simulink model "BaseEngineController_A02_bak"  (Simulink 7.7)
settings: Solver=FixedStepDiscrete  StartTime=0.0  StopTime=10.0  RelTol=1e-3
PreLoadFcn:
  | try, eval([bdroot '_setup']); catch, eval('model_setup', ''); end
InitFcn:
  | try, eval([bdroot '_setup']); catch, eval('model_setup', ''); end
undefined symbols (not defined in the file or in its folder): ECU555, High, motohawk_build_version, motohawk_get_faults, motohawk_trunc_name, motohawk_vardec_config_group, motohawk_vardec_path, True
libraries: MotoHawk_lib x1145, AutoDoc_lib x786, motohawk_control_primitives_lib x114, motohawk_engine_lib x19, motohawk_annotations_lib x12, simulink x11, simulink_extras x1
(part 4 of 23)

== system BaseEngineController_A02_bak/Foreground/Control/ActuatorCharacterization/Injector Characterization ==
  FPC [mg] : Inport
  FuelMult : Inport  Port=2
  BaroPress [kPa] : Inport  Port=3
  MAP [kPa] : Inport  Port=4
  SysVolt09Idx : Inport  Port=5
  RPM : Inport  Port=6
  Constant : Constant  Value=60*1000*2
  DeltaPressureIndex : Subsystem
  Divide : Product  Inputs=*/
  Documentation_Calibrations_Probes_Block : AutoDoc_lib/Documentation_Calibrations_Probes_Block
  Documentation_Left_Border : AutoDoc_lib/Documentation_Left_Border  layout=Portrait
  Documentation_Right_Border : AutoDoc_lib/Documentation_Right_Border  layout=Portrait
  Documentation_Title_Block : AutoDoc_lib/Documentation_Title_Block
  Fuel Inj Offsets : Subsystem
  Injector Gain [ms/mg] : MotoHawk_lib/Calibration & Probing Blocks/motohawk_calibration  nam='InjGain'  val=0.351  storage=Calibration  show_more=on  name_source=Use Parameter  data_type=Inherit from 'Default Value'  read_level=1  write_level=1  ovr_cals=on  view_as=Number  enum_struct={'Disabled', 'Enabled'}  vector_style=Wide Row  use_help_units=on  help='Gain value used in the linear transformation of FPC to mechanical pulsewidth.'  units='ms/mg'  use_header_enum=off  row_header_enum={}  col_header_enum={}  use_min_max=off  min_val=0  max_val=inf  use_precision=off  precision='2.4'  gain=1  offset=0  exp=1  use_mototune_group=on  mototune_group='Actuators | Injectors | Injector Config'
  Injector Offset [ms] : MotoHawk_lib/Calibration & Probing Blocks/motohawk_calibration  nam='InjOffset'  val=-0.05  storage=Calibration  show_more=on  name_source=Use Parameter  data_type=Inherit from 'Default Value'  read_level=1  write_level=1  ovr_cals=on  view_as=Number  enum_struct={'Disabled', 'Enabled'}  vector_style=Wide Row  use_help_units=on  help='Offset value used in the linear transformation of FPC to mechanical pulsewidth.'  units='ms'  use_header_enum=off  row_header_enum={}  col_header_enum={}  use_min_max=off  min_val=-inf  max_val=inf  use_precision=off  precision='3.4'  gain=1  offset=0  exp=1  use_mototune_group=on  mototune_group='Actuators | Injectors | Injector Config'
  MinMax : MinMax  Inputs=2
  Mux1 : Mux  Inputs=3
  Product2 : Product  SaturateOnIntegerOverflow=on  InputSameDT=on  OutDataTypeStr=Inherit: Same as first input
  Product3 : Product  Inputs=3  SaturateOnIntegerOverflow=on  InputSameDT=on  OutDataTypeStr=Inherit: Same as first input
  Regulated Gauge Fuel Pressure [kPa] : MotoHawk_lib/Calibration & Probing Blocks/motohawk_calibration  nam='GaugeFuelPress'  val=343  storage=Calibration  show_more=on  name_source=Use Parameter  data_type=Inherit from 'Default Value'  read_level=1  write_level=1  ovr_cals=on  view_as=Number  enum_struct={'Disabled', 'Enabled'}  vector_style=Wide Row  use_help_units=on  help='Fuel pressure of fuel rail.'  units='kPa'  use_header_enum=off  row_header_enum={}  col_header_enum={}  use_min_max=on  min_val=0  max_val=inf  use_precision=off  precision=''  gain=1  offset=0  exp=1  use_mototune_group=on  mototune_group='Actuators | Injectors | Injector Config'
  Saturation : Saturate  UpperLimit=inf  LowerLimit=.01
  Sum : Sum  Inputs=|++  SaturateOnIntegerOverflow=on  InputSameDT=on
  Sum1 : Sum  Inputs=|+-  SaturateOnIntegerOverflow=on
  Sum2 : Sum  Inputs=|-+  SaturateOnIntegerOverflow=on
  motohawk_interpolation_1d1 : MotoHawk_lib/Lookup Tables/motohawk_interpolation_1d  nam='InjTurnOn'  table_data=[1.495 1.293 1.155 1.05 0.968 0.898 0.865 0.823 0.743]  show_more=on  data_type=Inherit from 'Table Data'  storage=NonAdaptive  read_level=1  write_level=1  ovr_cals=on  use_help=on  help='Injector turn on time.'  units='ms'  use_min_max=off  min_val=-inf  max_val=inf  use_precision=off  precision='2.3'  gain=1  offset=0  exp=1  use_links=off  prelookup_block=''  output_name=''  use_mototune_group=on  mototune_group='Actuators | Injectors'
  motohawk_interpolation_1d3 : MotoHawk_lib/Lookup Tables/motohawk_interpolation_1d  nam='InjdPComp'  table_data=[1 1 1 1 1 1 1 1 1]  show_more=on  data_type=Inherit from 'Table Data'  storage=NonAdaptive  read_level=1  write_level=1  ovr_cals=on  use_help=on  help='Injector characterization compenastion based on dP across injector.'  units=''  use_min_max=on  min_val=-inf  max_val=inf  use_precision=on  precision='2.3'  gain=1  offset=0  exp=1  use_links=off  prelookup_block=''  output_name=''  use_mototune_group=on  mototune_group='Actuators | Injectors'
  motohawk_interpolation_1d4 : MotoHawk_lib/Lookup Tables/motohawk_interpolation_1d  nam='InjTurnOff'  table_data=[0.585 0.583333 0.581667 0.58 0.579167 0.578333 0.576667 0.575833 0.575]  show_more=on  data_type=Inherit from 'Table Data'  storage=NonAdaptive  read_level=1  write_level=1  ovr_cals=on  use_help=on  help='Injector turn off time'  units='ms'  use_min_max=on  min_val=-inf  max_val=inf  use_precision=on  precision='2.3'  gain=1  offset=0  exp=1  use_links=off  prelookup_block=''  output_name=''  use_mototune_group=on  mototune_group='Actuators | Injectors'
  motohawk_probe1 : MotoHawk_lib/Calibration & Probing Blocks/motohawk_probe  nam='InjEPW'  show_more=on  name_source=Use Parameter  read_level=1  view_as=Number  enum_struct={'Disabled', 'Enabled'}  vector_style=Wide Row  use_help_units=on  help=''  units=''  use_header_enum=off  row_header_enum={}  col_header_enum={}  use_precision=off  precision=''  gain=1  offset=0  exp=1  use_mototune_group=on  mototune_group='Actuators | Injectors'
  motohawk_probe3 : MotoHawk_lib/Calibration & Probing Blocks/motohawk_probe  nam='InjMPW'  show_more=on  name_source=Use Parameter  read_level=1  view_as=Number  enum_struct={'Disabled', 'Enabled'}  vector_style=Wide Row  use_help_units=on  help=''  units=''  use_header_enum=off  row_header_enum={}  col_header_enum={}  use_precision=off  precision=''  gain=1  offset=0  exp=1  use_mototune_group=on  mototune_group='Actuators | Injectors'
  motohawk_probe4 : MotoHawk_lib/Calibration & Probing Blocks/motohawk_probe  nam='MaxAllowableMPW'  show_more=on  name_source=Use Parameter  read_level=1  view_as=Number  enum_struct={'Disabled', 'Enabled'}  vector_style=Wide Row  use_help_units=on  help=''  units=''  use_header_enum=off  row_header_enum={}  col_header_enum={}  use_precision=off  precision=''  gain=1  offset=0  exp=1  use_mototune_group=on  mototune_group='Actuators | Injectors'
  MPW [ms] : Outport
  EPWOffset [ms] : Outport  Port=2
  wires:
  DeltaPressureIndex/1(Index) -> motohawk_interpolation_1d3/1, motohawk_interpolation_1d4/1
  FPC [mg]/1 -> Fuel Inj Offsets/1(Fuel In)
  Injector Gain [ms/mg]/1 -> Product2/2
  Injector Offset [ms]/1 -> Sum/2
  SysVolt09Idx/1 -> motohawk_interpolation_1d1/1
  Sum/1 -> Product3/2
  motohawk_interpolation_1d1/1 -> Sum2/2
  motohawk_interpolation_1d4/1 -> Sum2/1
  Mux1/1 -> DeltaPressureIndex/1(Inputs)
  Regulated Gauge Fuel Pressure [kPa]/1 -> Mux1/1
  BaroPress [kPa]/1 -> Mux1/2
  MAP [kPa]/1 -> Mux1/3
  Sum2/1 -> EPWOffset [ms]/1, Sum1/2, motohawk_probe1/1
  Product2/1 -> Sum/1
  Fuel Inj Offsets/1(Fuel Out) -> Product2/1
  Constant/1 -> Divide/1
  Product3/1 -> MinMax/1
  MinMax/1 -> motohawk_probe3/1, MPW [ms]/1
  Sum1/1 -> MinMax/2, motohawk_probe4/1
  Divide/1 -> Sum1/1
  RPM/1 -> Saturation/1
  Saturation/1 -> Divide/2
  motohawk_interpolation_1d3/1 -> Product3/3
  FuelMult/1 -> Product3/1

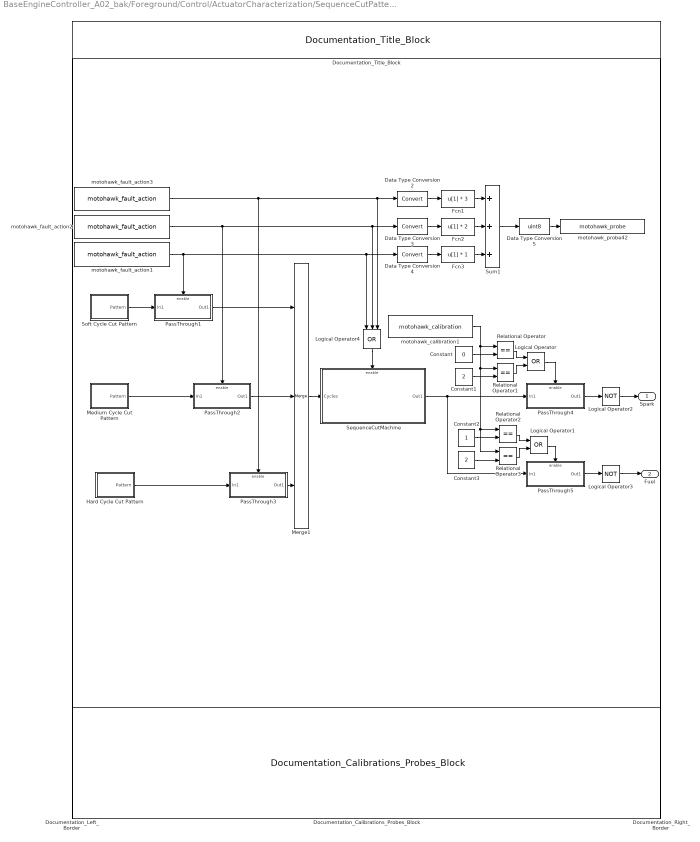
== system BaseEngineController_A02_bak/Foreground/Control/ActuatorCharacterization/SequenceCutPattern ==
  Constant : Constant  Value=0  OutDataTypeStr=Inherit: Inherit via back propagation  SampleTime=-1
  Constant1 : Constant  Value=2  OutDataTypeStr=Inherit: Inherit via back propagation  SampleTime=-1
  Constant2 : Constant  OutDataTypeStr=Inherit: Inherit via back propagation  SampleTime=-1
  Constant3 : Constant  Value=2  OutDataTypeStr=Inherit: Inherit via back propagation  SampleTime=-1
  Data Type Conversion2 : DataTypeConversion  SaturateOnIntegerOverflow=on  RndMeth=Zero
  Data Type Conversion3 : DataTypeConversion  SaturateOnIntegerOverflow=on  RndMeth=Zero
  Data Type Conversion4 : DataTypeConversion  SaturateOnIntegerOverflow=on  RndMeth=Zero
  Data Type Conversion5 : DataTypeConversion  OutDataTypeStr=uint8  SaturateOnIntegerOverflow=on  RndMeth=Zero
  Documentation_Calibrations_Probes_Block : AutoDoc_lib/Documentation_Calibrations_Probes_Block
  Documentation_Left_Border : AutoDoc_lib/Documentation_Left_Border  layout=Portrait
  Documentation_Right_Border : AutoDoc_lib/Documentation_Right_Border  layout=Portrait
  Documentation_Title_Block : AutoDoc_lib/Documentation_Title_Block
  Fcn1 : Fcn  Expr=u[1] * 3
  Fcn2 : Fcn  Expr=u[1] * 2
  Fcn3 : Fcn  Expr=u[1] * 1
  Hard Cycle Cut Pattern : Subsystem
  Logical Operator : Logic  Operator=OR
  Logical Operator1 : Logic  Operator=OR
  Logical Operator2 : Logic  Operator=NOT
  Logical Operator3 : Logic  Operator=NOT
  Logical Operator4 : Logic  Operator=OR  Inputs=3  AllPortsSameDT=off
  Medium Cycle Cut Pattern : Subsystem
  Merge1 : Merge  Inputs=3  AllowUnequalInputPortWidths=on
  PassThrough1 : Subsystem  TreatAsAtomicUnit=on
  PassThrough2 : Subsystem  TreatAsAtomicUnit=on
  PassThrough3 : Subsystem  TreatAsAtomicUnit=on
  PassThrough4 : Subsystem  TreatAsAtomicUnit=on
  PassThrough5 : Subsystem  TreatAsAtomicUnit=on
  Relational Operator{,1..3} (4 alike) : RelationalOperator  Operator===  InputSameDT=on
  SequenceCutMachine : Subsystem  TreatAsAtomicUnit=on
      mask Description:
      | When enabled, this block cycles though the 4 input patterns of cylinder disables that can be applied to fuel or spark.
      | 
      | The output should act on the enable inputs for MotoHawk EST and FInj blocks.  Outputs are reset when disabled.
      | 
      | Calibration arrays should be cast as boolean, and can be set up for any cylinder configuration.
      mask Display:
      | patch([0 0 1 1], [0 0.075 0.075 0], [230/255 0/255 0/255])
      | patch([0 0 1 1], [0.025 0.05 0.05 0.025], [150/255 0/255 0/255])
      | patch([0 0 1 1], [0 0.025 0.025 0], [0/255 0/255 0/255])
      | 
      | disp('SEQUENCE DISABLE\nPATTERN');
  Soft Cycle Cut Pattern : Subsystem
  Sum1 : Sum  Inputs=+++  SaturateOnIntegerOverflow=on
  motohawk_calibration1 : MotoHawk_lib/Calibration & Probing Blocks/motohawk_calibration  nam='RevLimitType'  val=2  storage=Calibration  show_more=on  name_source=Use Parameter  data_type=int8  read_level=1  write_level=1  ovr_cals=on  view_as=Enumeration  enum_struct={'Cut Spark', 'Cut Fuel', 'Cut Spark and Fuel'}  vector_style=Wide Row  use_help_units=on  help='Define the type of sequence to be disable when in Rev Limit'  units=''  use_header_enum=off  row_header_enum={}  col_header_enum={}  use_min_max=off  min_val=-inf  max_val=inf  use_precision=off  precision=''  gain=1  offset=0  exp=1  use_mototune_group=on  mototune_group='Diagnostics & Protection | RevLimitConfig'
  motohawk_fault_action1 : MotoHawk_lib/Fault Management Blocks/motohawk_fault_action  nam='SoftRevLimit'  output_status=on  eval_nam=SoftRevLimit
  motohawk_fault_action2 : MotoHawk_lib/Fault Management Blocks/motohawk_fault_action  nam='MediumRevLimit'  output_status=on  eval_nam=MediumRevLimit
  motohawk_fault_action3 : MotoHawk_lib/Fault Management Blocks/motohawk_fault_action  nam='HardRevLimit'  output_status=on  eval_nam=HardRevLimit
  motohawk_probe42 : MotoHawk_lib/Calibration & Probing Blocks/motohawk_probe  nam='RevLimiterState'  show_more=on  name_source=Use Parameter  read_level=1  view_as=Enumeration  enum_struct={'Disabled', 'Soft', 'Medium', 'Hard'}  vector_style=Wide Row  use_help_units=off  help=''  units=''  use_header_enum=off  row_header_enum={}  col_header_enum={}  use_precision=off  precision=''  gain=1  offset=0  exp=1  use_mototune_group=on  mototune_group='Diagnostics & Protection | RevLimit'
  Spark : Outport
  Fuel : Outport  Port=2
  wires:
  Logical Operator/1 -> PassThrough4/Enable
  PassThrough4/1(Out1) -> Logical Operator2/1
  PassThrough5/1(Out1) -> Logical Operator3/1
  Logical Operator1/1 -> PassThrough5/Enable
  Relational Operator/1 -> Logical Operator/1
  Relational Operator1/1 -> Logical Operator/2
  motohawk_calibration1/1 -> Relational Operator/1, Relational Operator1/1, Relational Operator2/1, Relational Operator3/1
  Constant/1 -> Relational Operator/2
  Constant1/1 -> Relational Operator1/2
  Constant2/1 -> Relational Operator2/2
  Constant3/1 -> Relational Operator3/2
  Relational Operator2/1 -> Logical Operator1/1
  Relational Operator3/1 -> Logical Operator1/2
  Logical Operator2/1 -> Spark/1
  Logical Operator3/1 -> Fuel/1
  SequenceCutMachine/1(Out1) -> PassThrough4/1(In1), PassThrough5/1(In1)
  Merge1/1 -> SequenceCutMachine/1(Cycles)
  PassThrough1/1(Out1) -> Merge1/1
  motohawk_fault_action1/1 -> PassThrough1/Enable, Logical Operator4/1, Data Type Conversion4/1
  Medium Cycle Cut Pattern/1(Pattern) -> PassThrough2/1(In1)
  PassThrough2/1(Out1) -> Merge1/2
  motohawk_fault_action2/1 -> PassThrough2/Enable, Logical Operator4/2, Data Type Conversion3/1
  Hard Cycle Cut Pattern/1(Pattern) -> PassThrough3/1(In1)
  PassThrough3/1(Out1) -> Merge1/3
  motohawk_fault_action3/1 -> PassThrough3/Enable, Logical Operator4/3, Data Type Conversion2/1
  Logical Operator4/1 -> SequenceCutMachine/Enable
  Soft Cycle Cut Pattern/1(Pattern) -> PassThrough1/1(In1)
  Data Type Conversion2/1 -> Fcn1/1
  Data Type Conversion3/1 -> Fcn2/1
  Data Type Conversion4/1 -> Fcn3/1
  Fcn1/1 -> Sum1/1
  Fcn2/1 -> Sum1/2
  Fcn3/1 -> Sum1/3
  Sum1/1 -> Data Type Conversion5/1
  Data Type Conversion5/1 -> motohawk_probe42/1

== system BaseEngineController_A02_bak/Foreground/Control/ActuatorCharacterization/Spark Advance to Start Angle ==
  Stop Angle [degCA] : Inport
  RPM : Inport  Port=2
  PulseWidth [ms] : Inport  Port=3
  Trigger : TriggerPort  TriggerType=function-call
  Documentation_Calibrations_Probes_Block : AutoDoc_lib/Documentation_Calibrations_Probes_Block
  Documentation_Left_Border : AutoDoc_lib/Documentation_Left_Border  layout=Portrait
  Documentation_Right_Border : AutoDoc_lib/Documentation_Right_Border  layout=Portrait
  Documentation_Title_Block : AutoDoc_lib/Documentation_Title_Block
  Product1 : Product  Inputs=**/  SaturateOnIntegerOverflow=on  InputSameDT=on  OutDataTypeStr=Inherit: Same as first input
  Product2 : Product  Inputs=**  SaturateOnIntegerOverflow=on  InputSameDT=on  OutDataTypeStr=Inherit: Same as first input
  Sum : Sum  Inputs=|++  SaturateOnIntegerOverflow=on  InputSameDT=on
  deg/rev : Constant  Value=360
  min/msec : Constant  Value=60000
  Start Angle [degCA] : Outport
  wires:
  Sum/1 -> Start Angle [degCA]/1
  RPM/1 -> Product1/1
  min/msec/1 -> Product1/3
  deg/rev/1 -> Product1/2
  PulseWidth [ms]/1 -> Product2/2  "PulseWidth [ms]"
  Product1/1 -> Product2/1  "deg/ms"
  Product2/1 -> Sum/2  "PulseWidth [degCA]"
  Stop Angle [degCA]/1 -> Sum/1

== system BaseEngineController_A02_bak/Foreground/Control/ActuatorCharacterization/Spark Coil Characterization ==
  SparkEnergy [mJ] : Inport
  SysVolt09Idx : Inport  Port=2
  Trigger : TriggerPort  TriggerType=function-call
  Constant : Constant  Value=1000
  Constant1 : Constant  Value=0  OutDataTypeStr=Inherit: Inherit via back propagation
  Documentation_Calibrations_Probes_Block : AutoDoc_lib/Documentation_Calibrations_Probes_Block
  Documentation_Left_Border : AutoDoc_lib/Documentation_Left_Border  layout=Portrait
  Documentation_Right_Border : AutoDoc_lib/Documentation_Right_Border  layout=Portrait
  Documentation_Title_Block : AutoDoc_lib/Documentation_Title_Block
  Only Enable Spark when Energy is Above Zero : RelationalOperator  Operator=>  ZeroCross=off  InputSameDT=on
  Product : Product  Inputs=*/  SaturateOnIntegerOverflow=on  OutDataTypeStr=Inherit: Same as first input
  Sum : Sum  Inputs=|++  SaturateOnIntegerOverflow=on
  motohawk_calibration : MotoHawk_lib/Calibration & Probing Blocks/motohawk_calibration  nam='CoilDrvrDlyTime'  val=0.004  storage=Calibration  show_more=on  name_source=Use Parameter  data_type=Inherit from 'Default Value'  read_level=1  write_level=1  ovr_cals=on  view_as=Number  enum_struct={'Disabled', 'Enabled'}  vector_style=Wide Row  use_help_units=on  help='Coil driver delay time between receipt of EST signal and initiation of coil dwell.'  units='ms'  use_header_enum=off  row_header_enum={}  col_header_enum={}  use_min_max=off  min_val=-inf  max_val=inf  use_precision=off  precision='2.3'  gain=1  offset=0  exp=1  use_mototune_group=on  mototune_group='Actuators | Coils | Coils Config'
  motohawk_interpolation_2d1 : MotoHawk_lib/Lookup Tables/motohawk_interpolation_2d  nam='CoilDwell'  table_data=ones(9)*2  show_more=on  data_type=Inherit from 'Table Data'  storage=NonAdaptive  read_level=1  write_level=1  ovr_cals=on  use_help=on  help='Required spark dwell to give the desired spark energy.'  units='ms'  use_min_max=on  min_val=-inf  max_val=inf  use_precision=on  precision=''  gain=1  offset=0  exp=1  use_links=off  row_prelookup_block=''  col_prelookup_block=''  output_name=''  use_mototune_group=on  mototune_group='Actuators | Coils'
  motohawk_override_rel : MotoHawk_lib/Calibration & Probing Blocks/motohawk_override_rel  SystemSampleTime=-1  parent_name='SparkDwell'  show_more=on  name_source=Use Parameter  parent_read_level=1  parent_override_level=1  use_help_units=off  parent_help=''  parent_units=''  use_min_max=off  parent_min=-inf  parent_max=inf  use_precision=off  parent_precision=''  parent_gain=1  parent_offset=0  parent_exp=1  use_mototune_group=on  parent_group='Actuators|Coils'
  motohawk_prelookup : MotoHawk_lib/Lookup Tables/motohawk_prelookup  nam='SpkEnergy09'  breakpoint_data=[10 19 29 38 48 57 66 76 86]  show_more=on  data_type=Inherit from 'Table Data'  storage=Constant  read_level=1  write_level=1  ovr_cals=on  use_help=on  help=''  units=''  use_min_max=off  min_val=-inf  max_val=inf  use_precision=on  precision=''  gain=1  offset=0  exp=1  use_links=off  input_name=''  use_mototune_group=on  mototune_group='Indexes'
  motohawk_probe : MotoHawk_lib/Calibration & Probing Blocks/motohawk_probe  nam='SparkDwell'  show_more=on  name_source=Use Parameter  read_level=1  view_as=Number  enum_struct={'Disabled', 'Enabled'}  vector_style=Wide Row  use_help_units=on  help='Total electrical spark dwell time'  units='ms'  use_header_enum=off  row_header_enum={}  col_header_enum={}  use_precision=off  precision=''  gain=1  offset=0  exp=1  use_mototune_group=on  mototune_group='Actuators|Coils'
  motohawk_probe1 : MotoHawk_lib/Calibration & Probing Blocks/motohawk_probe  nam='SparkEnergyMonitor'  show_more=on  name_source=Use Parameter  read_level=1  view_as=Number  enum_struct={'Disabled', 'Enabled'}  vector_style=Wide Row  use_help_units=on  help='Commanded spark energy, before conversion to dwell time'  units='mJ'  use_header_enum=off  row_header_enum={}  col_header_enum={}  use_precision=off  precision=''  gain=1  offset=0  exp=1  use_mototune_group=on  mototune_group='Actuators|Coils'
  motohawk_probe2 : MotoHawk_lib/Calibration & Probing Blocks/motohawk_probe  nam='SparkEnabled'  show_more=on  name_source=Use Parameter  read_level=1  view_as=Number  enum_struct={'Disabled', 'Enabled'}  vector_style=Wide Row  use_help_units=on  help='Enabled when spark energy is greater than zero'  units='boolean'  use_header_enum=off  row_header_enum={}  col_header_enum={}  use_precision=off  precision=''  gain=1  offset=0  exp=1  use_mototune_group=on  mototune_group='Actuators|Coils'
  SparkDwell [ms] : Outport
  SparkEnable [bool] : Outport  Port=2
  wires:
  SysVolt09Idx/1 -> motohawk_interpolation_2d1/2  "SysVolt10Idx"
  motohawk_prelookup/1 -> motohawk_interpolation_2d1/1  "SpkEnergy09Idx"
  SparkEnergy [mJ]/1 -> motohawk_probe1/1, Only Enable Spark when Energy is Above Zero/1, motohawk_prelookup/1
  Sum/1 -> motohawk_override_rel/1
  motohawk_calibration/1 -> Product/1
  Product/1 -> Sum/2
  Constant/1 -> Product/2
  Only Enable Spark when Energy is Above Zero/1 -> SparkEnable [bool]/1, motohawk_probe2/1
  Constant1/1 -> Only Enable Spark when Energy is Above Zero/2
  motohawk_override_rel/1 -> motohawk_probe/1, SparkDwell [ms]/1
  motohawk_interpolation_2d1/1 -> Sum/1

== system BaseEngineController_A02_bak/Foreground/Control/Controller/Crank ==
  Inputs : Inport
  Enable : EnablePort  StatesWhenEnabling=reset
  Bus Creator2 : BusCreator  Inputs=11
  Bus Selector : BusSelector  OutputSignals=Raw.RPM,Raw.NonLinearSensors.ECT,RawProcessed.APP_Raw,RawProcessed.TPS
  Cals_and_Probes_Page : AutoDoc_lib/Cals_and_Probes_Page  SystemSampleTime=-1
  CrankFueling : Subsystem
  Documentation_Calibrations_Probes_Block : AutoDoc_lib/Documentation_Calibrations_Probes_Block
  Documentation_Left_Border : AutoDoc_lib/Documentation_Left_Border  layout=Portrait
  Documentation_Right_Border : AutoDoc_lib/Documentation_Right_Border  layout=Portrait
  Documentation_Title_Block : AutoDoc_lib/Documentation_Title_Block
  EGR : Constant  Value=0  OutDataTypeStr=double
  Mult : Constant  OutDataTypeStr=double
  O2 Heater : Constant  OutDataTypeStr=boolean
  OILP : Constant  OutDataTypeStr=boolean
  ON : Constant  OutDataTypeStr=boolean
  Zero-Order Hold{1..11} (11 alike) : ZeroOrderHold  SampleTime=-1
  motohawk_interpolation_1d1 : MotoHawk_lib/Lookup Tables/motohawk_interpolation_1d  nam='CrankAirflowSetPt'  table_data=[34 34 34 31 30]  show_more=on  data_type=Inherit from 'Table Data'  storage=NonAdaptive  read_level=1  write_level=1  ovr_cals=on  use_help=on  help='ETC setpoint during cranking.'  units='%'  use_min_max=off  min_val=-inf  max_val=inf  use_precision=off  precision=''  gain=1  offset=0  exp=1  use_links=off  prelookup_block=''  output_name=''  use_mototune_group=on  mototune_group=motohawk_vardec_path(gcb)
  motohawk_interpolation_1d2 : MotoHawk_lib/Lookup Tables/motohawk_interpolation_1d  nam='CrankSpark'  table_data=[1 1 1 1 1]  show_more=on  data_type=Inherit from 'Table Data'  storage=NonAdaptive  read_level=1  write_level=1  ovr_cals=on  use_help=on  help='Spark adv during cranking.'  units='degBTDC'  use_min_max=on  min_val=-100  max_val=100  use_precision=on  precision=''  gain=1  offset=0  exp=1  use_links=off  prelookup_block=''  output_name=''  use_mototune_group=on  mototune_group=motohawk_vardec_path(gcb)
  motohawk_interpolation_1d4 : MotoHawk_lib/Lookup Tables/motohawk_interpolation_1d  nam='CrankSOI'  table_data=[500 500 500 500 500]  show_more=on  data_type=Inherit from 'Table Data'  storage=NonAdaptive  read_level=1  write_level=1  ovr_cals=on  use_help=on  help='Start of injection during cranking.'  units='degBTDC'  use_min_max=on  min_val=-920  max_val=920  use_precision=on  precision=''  gain=1  offset=0  exp=1  use_links=off  prelookup_block=''  output_name=''  use_mototune_group=on  mototune_group=motohawk_vardec_path(gcb)
  motohawk_interpolation_1d5 : MotoHawk_lib/Lookup Tables/motohawk_interpolation_1d  nam='CrankMakeUpEOI'  table_data=[200 200 200 200 200]  show_more=on  data_type=Inherit from 'Table Data'  storage=NonAdaptive  read_level=1  write_level=1  ovr_cals=on  use_help=on  help='Start of injection during cranking.'  units='degBTDC'  use_min_max=on  min_val=-920  max_val=920  use_precision=on  precision=''  gain=1  offset=0  exp=1  use_links=off  prelookup_block=''  output_name=''  use_mototune_group=on  mototune_group=motohawk_vardec_path(gcb)
  motohawk_interpolation_2d1 : MotoHawk_lib/Lookup Tables/motohawk_interpolation_2d  nam='CrankSpkEnergy'  table_data=[80 72.5 65 57 50;80 72.5 65 57.5 50;80 72.5 65 57.5 50;80 72.5 65 57.5 50;80 72.5 65 57.5 50]  show_more=on  data_type=Inherit from 'Table Data'  storage=NonAdaptive  read_level=1  write_level=1  ovr_cals=on  use_help=on  help='Spark energy during cranking.'  units='MJ'  use_min_max=on  min_val=0  max_val=inf  use_precision=on  precision=''  gain=1  offset=0  exp=1  use_links=off  row_prelookup_block=''  col_prelookup_block=''  output_name=''  use_mototune_group=on  mototune_group=motohawk_vardec_path(gcb)
  motohawk_prelookup : MotoHawk_lib/Lookup Tables/motohawk_prelookup  nam='CrankRPM05'  breakpoint_data=[0 100 200 400 500]  show_more=on  data_type=Inherit from 'Table Data'  storage=Constant  read_level=1  write_level=1  ovr_cals=on  use_help=on  help='RPM pre-lookup for crank maps'  units='RPM'  use_min_max=off  min_val=-inf  max_val=inf  use_precision=off  precision=''  gain=1  offset=0  exp=1  use_links=on  input_name=''  use_mototune_group=on  mototune_group=motohawk_vardec_path(gcb)
  motohawk_prelookup1 : MotoHawk_lib/Lookup Tables/motohawk_prelookup  nam='CrankECT05'  breakpoint_data=[-40 -3.75 32.5 68.75 105]  show_more=on  data_type=Inherit from 'Table Data'  storage=Constant  read_level=1  write_level=1  ovr_cals=on  use_help=on  help='ECT pre-lookup for crank maps.'  units='degC'  use_min_max=off  min_val=-inf  max_val=inf  use_precision=off  precision=''  gain=1  offset=0  exp=1  use_links=on  input_name='ECT'  use_mototune_group=on  mototune_group=motohawk_vardec_path(gcb)
  motohawk_replicate : MotoHawk_lib/Extra Development Blocks/motohawk_replicate  output_width=-1
  motohawk_replicate1 : MotoHawk_lib/Extra Development Blocks/motohawk_replicate  output_width=-1
  Actuators : Outport
  wires:
  Inputs/1 -> Bus Selector/1
  motohawk_prelookup1/1 -> CrankFueling/2(ECTIdx), motohawk_interpolation_1d1/1, motohawk_interpolation_2d1/1
  motohawk_prelookup/1 -> motohawk_interpolation_1d4/1, motohawk_interpolation_1d5/1, motohawk_interpolation_1d2/1, motohawk_interpolation_2d1/2, CrankFueling/1(RPMIdx)
  Bus Selector/2 -> motohawk_prelookup1/1  "<ECT>"
  Bus Selector/1 -> motohawk_prelookup/1  "<RPM>"
  motohawk_interpolation_1d2/1 -> motohawk_replicate1/1
  CrankFueling/1(FPC) -> motohawk_replicate/1
  Zero-Order Hold6/1 -> Bus Creator2/1  "FPC"
  Zero-Order Hold5/1 -> Bus Creator2/2  "SOI"
  ON/1 -> Zero-Order Hold7/1
  motohawk_interpolation_1d1/1 -> Zero-Order Hold9/1
  motohawk_interpolation_1d4/1 -> Zero-Order Hold5/1
  Zero-Order Hold4/1 -> Bus Creator2/4  "SparkEnergy"
  Zero-Order Hold3/1 -> Bus Creator2/5  "SparkAdv"
  Zero-Order Hold9/1 -> Bus Creator2/6  "Throttle"
  Zero-Order Hold7/1 -> Bus Creator2/7  "FUELP"
  motohawk_replicate/1 -> Zero-Order Hold6/1
  motohawk_interpolation_1d5/1 -> Zero-Order Hold8/1
  Zero-Order Hold8/1 -> Bus Creator2/3  "EOI"
  motohawk_replicate1/1 -> Zero-Order Hold3/1
  Bus Selector/3 -> CrankFueling/3(APP)  "<APP_Raw>"
  Zero-Order Hold10/1 -> Bus Creator2/8  "FuelMult"
  Mult/1 -> Zero-Order Hold10/1
  motohawk_interpolation_2d1/1 -> Zero-Order Hold4/1
  Bus Selector/4 -> CrankFueling/4(TPS)  "<TPS>"
  Bus Creator2/1 -> Actuators/1
  EGR/1 -> Zero-Order Hold1/1
  Zero-Order Hold1/1 -> Bus Creator2/9  "EGR"
  OILP/1 -> Zero-Order Hold2/1
  Zero-Order Hold2/1 -> Bus Creator2/10  "OILP"
  O2 Heater/1 -> Zero-Order Hold11/1
  Zero-Order Hold11/1 -> Bus Creator2/11  "O2 Heater"

== system BaseEngineController_A02_bak/Foreground/Control/Controller/Hybrid ==
  In1 : Inport
  Bus Creator : BusCreator
  Bus Selector : BusSelector  OutputSignals=Raw.CAN Inputs.112.Genset Enable,Raw.CAN Inputs.112.Load Enable,Raw.RPM
  Product : Product  RndMeth=Zero
  Product1 : Product  RndMeth=Zero
  Remy Control : Stateflow chart  TreatAsAtomicUnit=on
  Switch : Switch  Criteria=u2 > Threshold
  Time Since Enabled (With Input)1 : motohawk_control_primitives_lib/Time Since Enabled (With Input)1  SystemSampleTime=-1  nam='ThrottleRampTime'  samp_time=-1  init=0  upperlim=16000
  Time Since Enabled (With Input)2 : motohawk_control_primitives_lib/Time Since Enabled (With Input)1  SystemSampleTime=-1  nam='TorqueRampTime'  samp_time=-1  init=0  upperlim=16000
  motohawk_calibration : MotoHawk_lib/Calibration & Probing Blocks/motohawk_calibration  nam='Crank_Time'  val=600  storage=Calibration  show_more=on  name_source=Use Parameter  data_type=Inherit from 'Default Value'  read_level=1  write_level=1  ovr_cals=on  view_as=Number  enum_struct={'Disabled', 'Enabled'}  vector_style=Wide Row  use_help_units=on  help=''  units='s'  use_header_enum=off  row_header_enum={}  col_header_enum={}  use_min_max=off  min_val=-inf  max_val=inf  use_precision=on  precision=''  gain=1/200  offset=0  exp=1  use_mototune_group=off  mototune_group=motohawk_vardec_path(gcb)
  motohawk_calibration1 : MotoHawk_lib/Calibration & Probing Blocks/motohawk_calibration  nam='Caught_RPM'  val=600  storage=Calibration  show_more=on  name_source=Use Parameter  data_type=Inherit from 'Default Value'  read_level=1  write_level=1  ovr_cals=on  view_as=Number  enum_struct={'Disabled', 'Enabled'}  vector_style=Wide Row  use_help_units=on  help=''  units='RPM'  use_header_enum=off  row_header_enum={}  col_header_enum={}  use_min_max=off  min_val=-inf  max_val=inf  use_precision=off  precision=''  gain=1  offset=0  exp=1  use_mototune_group=off  mototune_group=motohawk_vardec_path(gcb)
  motohawk_calibration2 : MotoHawk_lib/Calibration & Probing Blocks/motohawk_calibration  nam='Cranking_Torque'  val=15  storage=Calibration  show_more=on  name_source=Use Parameter  data_type=Inherit from 'Default Value'  read_level=1  write_level=1  ovr_cals=on  view_as=Number  enum_struct={'Disabled', 'Enabled'}  vector_style=Wide Row  use_help_units=on  help=''  units='nm'  use_header_enum=off  row_header_enum={}  col_header_enum={}  use_min_max=off  min_val=-inf  max_val=inf  use_precision=off  precision=''  gain=1  offset=0  exp=1  use_mototune_group=off  mototune_group=motohawk_vardec_path(gcb)
  motohawk_calibration4 : MotoHawk_lib/Calibration & Probing Blocks/motohawk_calibration  nam='Max_Cranking_RPM'  val=400  storage=Calibration  show_more=on  name_source=Use Parameter  data_type=Inherit from 'Default Value'  read_level=1  write_level=1  ovr_cals=on  view_as=Number  enum_struct={'Disabled', 'Enabled'}  vector_style=Wide Row  use_help_units=on  help=''  units='RPM'  use_header_enum=off  row_header_enum={}  col_header_enum={}  use_min_max=off  min_val=-inf  max_val=inf  use_precision=off  precision=''  gain=1  offset=0  exp=1  use_mototune_group=off  mototune_group=motohawk_vardec_path(gcb)
  motohawk_calibration5 : MotoHawk_lib/Calibration & Probing Blocks/motohawk_calibration  nam='Max_Generating_RPM'  val=3600  storage=Calibration  show_more=on  name_source=Use Parameter  data_type=Inherit from 'Default Value'  read_level=1  write_level=1  ovr_cals=on  view_as=Number  enum_struct={'Disabled', 'Enabled'}  vector_style=Wide Row  use_help_units=on  help=''  units='RPM'  use_header_enum=off  row_header_enum={}  col_header_enum={}  use_min_max=off  min_val=-inf  max_val=inf  use_precision=off  precision=''  gain=1  offset=0  exp=1  use_mototune_group=off  mototune_group=motohawk_vardec_path(gcb)
  motohawk_calibration6 : MotoHawk_lib/Calibration & Probing Blocks/motohawk_calibration  nam='Crank_Wait_Before_Retry'  val=600  storage=Calibration  show_more=on  name_source=Use Parameter  data_type=Inherit from 'Default Value'  read_level=1  write_level=1  ovr_cals=on  view_as=Number  enum_struct={'Disabled', 'Enabled'}  vector_style=Wide Row  use_help_units=on  help=''  units='s'  use_header_enum=off  row_header_enum={}  col_header_enum={}  use_min_max=off  min_val=-inf  max_val=inf  use_precision=on  precision=''  gain=1/200  offset=0  exp=1  use_mototune_group=off  mototune_group=motohawk_vardec_path(gcb)
  motohawk_data_def : MotoHawk_lib/Data Storage Blocks/motohawk_data_def  nam='APP'  container_nam=''  data=0  typ=double  struct_nam='MyStruct'  storage=Volatile  outputRef=off  use_vardec=on  window=Display  read_level=1  write_level=1  ovr_cals=on  view_as=Number  enum_struct={'Disabled', 'Enabled'}  vector_style=Wide Row  use_help_units=off  help=''  units=''  use_header_enum=off  row_header_enum=''  col_header_enum=''  use_min_max=off  min_val=-inf  max_val=inf  use_precision=off  precision=''  gain=1  offset=0  exp=1  use_mototune_group=off  mototune_group=motohawk_vardec_path(gcb)  eval_name=MyData  data_stores=motohawk_sfun_data_def_data_stores  struct_instances=motohawk_sfun_data_def_struct_instances  vardecs=motohawk_sfun_data_def_vardecs
  motohawk_data_write : MotoHawk_lib/Data Storage Blocks/motohawk_data_write  source=Lookup By Name  structure=Scalar  operation=Write entire data structure at once  sz_vec=9  sz_row=2  sz_col=9  typ=double  nam='APP'  checkNull=on  write_read_only=off  struct_inst_name=''  struct_name='MyStruct'
  motohawk_interpolation_1d : MotoHawk_lib/Lookup Tables/motohawk_interpolation_1d  nam='APP_Interpolation'  table_data=[0,0,0,0,0,0,0]  show_more=on  data_type=Inherit from 'Table Data'  storage=NonAdaptive  read_level=1  write_level=1  ovr_cals=on  use_help=on  help=''  units='us'  use_min_max=on  min_val=0  max_val=inf  use_precision=off  precision=''  gain=1  offset=0  exp=1  use_links=off  prelookup_block=''  output_name=''  use_mototune_group=off  mototune_group=motohawk_vardec_path(gcb)
  motohawk_interpolation_1d1 : MotoHawk_lib/Lookup Tables/motohawk_interpolation_1d  nam='TQ_Interpolation'  table_data=[0,0,0,0,0,0,0]  show_more=on  data_type=Inherit from 'Table Data'  storage=NonAdaptive  read_level=1  write_level=1  ovr_cals=on  use_help=on  help=''  units='us'  use_min_max=on  min_val=0  max_val=inf  use_precision=off  precision=''  gain=1  offset=0  exp=1  use_links=off  prelookup_block=''  output_name=''  use_mototune_group=off  mototune_group=motohawk_vardec_path(gcb)
  motohawk_prelookup : MotoHawk_lib/Lookup Tables/motohawk_prelookup  nam='APP_Prelookup'  breakpoint_data=[0:0.5:3]  show_more=on  data_type=Inherit from 'Table Data'  storage=Constant  read_level=1  write_level=1  ovr_cals=on  use_help=on  help=''  units=''  use_min_max=off  min_val=-inf  max_val=inf  use_precision=off  precision=''  gain=1  offset=0  exp=1  use_links=off  input_name=''  use_mototune_group=off  mototune_group=motohawk_vardec_path(gcb)
  motohawk_prelookup1 : MotoHawk_lib/Lookup Tables/motohawk_prelookup  nam='TQ_Prelookup'  breakpoint_data=[0:0.5:3]  show_more=on  data_type=Inherit from 'Table Data'  storage=Constant  read_level=1  write_level=1  ovr_cals=on  use_help=on  help=''  units=''  use_min_max=off  min_val=-inf  max_val=inf  use_precision=off  precision=''  gain=1  offset=0  exp=1  use_links=off  input_name=''  use_mototune_group=off  mototune_group=motohawk_vardec_path(gcb)
  motohawk_probe : MotoHawk_lib/Calibration & Probing Blocks/motohawk_probe  nam='cranking_Prb'  show_more=off  name_source=Use Parameter  read_level=1  view_as=Number  enum_struct={'Disabled', 'Enabled'}  vector_style=Wide Row  use_help_units=off  help=''  units=''  use_header_enum=off  row_header_enum={}  col_header_enum={}  use_precision=off  precision=''  gain=1  offset=0  exp=1  use_mototune_group=off  mototune_group=motohawk_vardec_path(gcb)
  motohawk_probe1 : MotoHawk_lib/Calibration & Probing Blocks/motohawk_probe  nam='idle_Prb'  show_more=off  name_source=Use Parameter  read_level=1  view_as=Number  enum_struct={'Disabled', 'Enabled'}  vector_style=Wide Row  use_help_units=off  help=''  units=''  use_header_enum=off  row_header_enum={}  col_header_enum={}  use_precision=off  precision=''  gain=1  offset=0  exp=1  use_mototune_group=off  mototune_group=motohawk_vardec_path(gcb)
  motohawk_probe2 : MotoHawk_lib/Calibration & Probing Blocks/motohawk_probe  nam='APPandTQ_Ramp_Prb'  show_more=off  name_source=Use Parameter  read_level=1  view_as=Number  enum_struct={'Disabled', 'Enabled'}  vector_style=Wide Row  use_help_units=off  help=''  units=''  use_header_enum=off  row_header_enum={}  col_header_enum={}  use_precision=off  precision=''  gain=1  offset=0  exp=1  use_mototune_group=off  mototune_group=motohawk_vardec_path(gcb)
  motohawk_probe3 : MotoHawk_lib/Calibration & Probing Blocks/motohawk_probe  nam='remyEn_Prb'  show_more=off  name_source=Use Parameter  read_level=1  view_as=Number  enum_struct={'Disabled', 'Enabled'}  vector_style=Wide Row  use_help_units=off  help=''  units=''  use_header_enum=off  row_header_enum={}  col_header_enum={}  use_precision=off  precision=''  gain=1  offset=0  exp=1  use_mototune_group=off  mototune_group=motohawk_vardec_path(gcb)
  motohawk_probe4 : MotoHawk_lib/Calibration & Probing Blocks/motohawk_probe  nam='maxRPM_Prb'  show_more=off  name_source=Use Parameter  read_level=1  view_as=Number  enum_struct={'Disabled', 'Enabled'}  vector_style=Wide Row  use_help_units=off  help=''  units=''  use_header_enum=off  row_header_enum={}  col_header_enum={}  use_precision=off  precision=''  gain=1  offset=0  exp=1  use_mototune_group=off  mototune_group=motohawk_vardec_path(gcb)
  motohawk_probe5 : MotoHawk_lib/Calibration & Probing Blocks/motohawk_probe  nam='motorTQ_Prb'  show_more=off  name_source=Use Parameter  read_level=1  view_as=Number  enum_struct={'Disabled', 'Enabled'}  vector_style=Wide Row  use_help_units=off  help=''  units=''  use_header_enum=off  row_header_enum={}  col_header_enum={}  use_precision=off  precision=''  gain=1  offset=0  exp=1  use_mototune_group=off  mototune_group=motohawk_vardec_path(gcb)
  motohawk_probe6 : MotoHawk_lib/Calibration & Probing Blocks/motohawk_probe  nam='generatingTQ_Prb'  show_more=off  name_source=Use Parameter  read_level=1  view_as=Number  enum_struct={'Disabled', 'Enabled'}  vector_style=Wide Row  use_help_units=off  help=''  units=''  use_header_enum=off  row_header_enum={}  col_header_enum={}  use_precision=off  precision=''  gain=1  offset=0  exp=1  use_mototune_group=off  mototune_group=motohawk_vardec_path(gcb)
  Out1 : Outport
  wires:
  In1/1 -> Bus Selector/1
  Bus Selector/1 -> Remy Control/1(genEnable)  "<Genset Enable>"
  Bus Selector/2 -> Remy Control/2(genLoad)  "<Load Enable>"
  Bus Selector/3 -> Remy Control/3(RPM)  "<RPM>"
  motohawk_calibration/1 -> Remy Control/4(crankTime)
  motohawk_calibration6/1 -> Remy Control/5(crankWait)
  motohawk_calibration1/1 -> Remy Control/6(caughtRPM)
  motohawk_calibration2/1 -> Remy Control/7(crankTQ)
  motohawk_calibration4/1 -> Remy Control/8(maxCrankRPM)
  motohawk_calibration5/1 -> Remy Control/9(maxMotorRPM)
  Remy Control/3(APPRamp) -> motohawk_probe2/1, Switch/2, Time Since Enabled (With Input)1/1, Product/2
  Remy Control/4(torqueRamp) -> Time Since Enabled (With Input)2/1, Product1/2
  Remy Control/1(cranking) -> motohawk_probe/1
  Remy Control/2(idle) -> motohawk_probe1/1
  motohawk_prelookup/1 -> motohawk_interpolation_1d/1
  motohawk_prelookup1/1 -> motohawk_interpolation_1d1/1
  Time Since Enabled (With Input)1/1 -> motohawk_prelookup/1
  Time Since Enabled (With Input)2/1 -> motohawk_prelookup1/1
  Bus Creator/1 -> Out1/1
  Remy Control/5(remyEn) -> Bus Creator/1, motohawk_probe3/1  "remyEn"
  Remy Control/6(maxRPM) -> motohawk_probe4/1, Bus Creator/2  "maxRPM"
  Remy Control/7(motorTQ) -> Bus Creator/3, motohawk_probe5/1  "motorTQ"
  Switch/1 -> Bus Creator/4, motohawk_probe6/1  "generatingTQ"
  Remy Control/8(generatingTQ) -> Switch/3
  motohawk_interpolation_1d/1 -> Product/1
  Product/1 -> motohawk_data_write/1
  motohawk_interpolation_1d1/1 -> Product1/1
  Product1/1 -> Switch/1

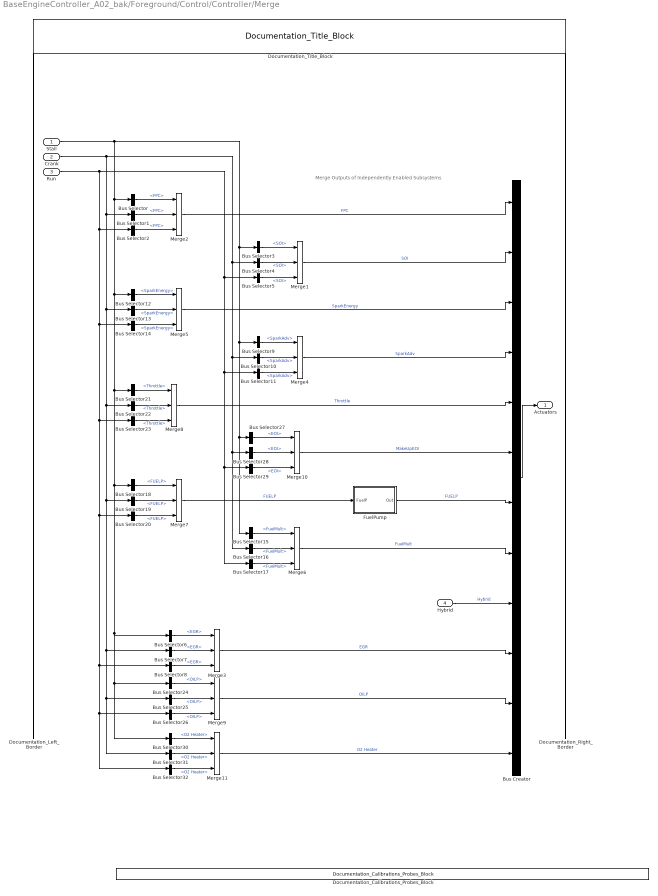
== system BaseEngineController_A02_bak/Foreground/Control/Controller/Merge ==
  note: Merge Outputs of Independently Enabled Subsystems
  Stall : Inport
  Crank : Inport  Port=2
  Run : Inport  Port=3
  Hybrid : Inport  Port=4
  Bus Creator : BusCreator  Inputs=12
  Bus Selector : BusSelector  OutputSignals=FPC
  Bus Selector1 : BusSelector  OutputSignals=FPC
  Bus Selector10 : BusSelector  OutputSignals=SparkAdv
  Bus Selector11 : BusSelector  OutputSignals=SparkAdv
  Bus Selector12 : BusSelector  OutputSignals=SparkEnergy
  Bus Selector13 : BusSelector  OutputSignals=SparkEnergy
  Bus Selector14 : BusSelector  OutputSignals=SparkEnergy
  Bus Selector15 : BusSelector  OutputSignals=FuelMult
  Bus Selector16 : BusSelector  OutputSignals=FuelMult
  Bus Selector17 : BusSelector  OutputSignals=FuelMult
  Bus Selector18 : BusSelector  OutputSignals=FUELP
  Bus Selector19 : BusSelector  OutputSignals=FUELP
  Bus Selector2 : BusSelector  OutputSignals=FPC
  Bus Selector20 : BusSelector  OutputSignals=FUELP
  Bus Selector21 : BusSelector  OutputSignals=Throttle
  Bus Selector22 : BusSelector  OutputSignals=Throttle
  Bus Selector23 : BusSelector  OutputSignals=Throttle
  Bus Selector24 : BusSelector  OutputSignals=OILP
  Bus Selector25 : BusSelector  OutputSignals=OILP
  Bus Selector26 : BusSelector  OutputSignals=OILP
  Bus Selector27 : BusSelector  OutputSignals=EOI
  Bus Selector28 : BusSelector  OutputSignals=EOI
  Bus Selector29 : BusSelector  OutputSignals=EOI
  Bus Selector3 : BusSelector  OutputSignals=SOI
  Bus Selector30 : BusSelector  OutputSignals=O2 Heater
  Bus Selector31 : BusSelector  OutputSignals=O2 Heater
  Bus Selector32 : BusSelector  OutputSignals=O2 Heater
  Bus Selector4 : BusSelector  OutputSignals=SOI
  Bus Selector5 : BusSelector  OutputSignals=SOI
  Bus Selector6 : BusSelector  OutputSignals=EGR
  Bus Selector7 : BusSelector  OutputSignals=EGR
  Bus Selector8 : BusSelector  OutputSignals=EGR
  Bus Selector9 : BusSelector  OutputSignals=SparkAdv
  Documentation_Calibrations_Probes_Block : AutoDoc_lib/Documentation_Calibrations_Probes_Block
  Documentation_Left_Border : AutoDoc_lib/Documentation_Left_Border  layout=Portrait
  Documentation_Right_Border : AutoDoc_lib/Documentation_Right_Border  layout=Portrait
  Documentation_Title_Block : AutoDoc_lib/Documentation_Title_Block
  FuelPump : Subsystem
  Merge{1..11} (11 alike) : Merge  Inputs=3
  Actuators : Outport
  wires:
  Bus Selector23/1 -> Merge8/3  "<Throttle>"
  Bus Selector22/1 -> Merge8/2  "<Throttle>"
  Bus Selector21/1 -> Merge8/1  "<Throttle>"
  Bus Selector20/1 -> Merge7/3  "<FUELP>"
  Bus Selector19/1 -> Merge7/2  "<FUELP>"
  Bus Selector18/1 -> Merge7/1  "<FUELP>"
  Merge7/1 -> FuelPump/1(FuelP)  "FUELP"
  Bus Selector14/1 -> Merge5/3  "<SparkEnergy>"
  Bus Selector13/1 -> Merge5/2  "<SparkEnergy>"
  Bus Selector12/1 -> Merge5/1  "<SparkEnergy>"
  Bus Selector11/1 -> Merge4/3  "<SparkAdv>"
  Bus Selector10/1 -> Merge4/2  "<SparkAdv>"
  Bus Selector9/1 -> Merge4/1  "<SparkAdv>"
  Bus Selector5/1 -> Merge1/3  "<SOI>"
  Bus Selector4/1 -> Merge1/2  "<SOI>"
  Bus Selector3/1 -> Merge1/1  "<SOI>"
  Run/1 -> Bus Selector11/1, Bus Selector29/1, Bus Selector17/1, Bus Selector5/1, Bus Selector2/1, Bus Selector14/1, Bus Selector23/1, Bus Selector20/1, Bus Selector8/1, Bus Selector26/1, Bus Selector32/1
  Crank/1 -> Bus Selector10/1, Bus Selector28/1, Bus Selector16/1, Bus Selector4/1, Bus Selector1/1, Bus Selector13/1, Bus Selector22/1, Bus Selector19/1, Bus Selector7/1, Bus Selector25/1, Bus Selector31/1
  Stall/1 -> Bus Selector9/1, Bus Selector27/1, Bus Selector15/1, Bus Selector3/1, Bus Selector/1, Bus Selector12/1, Bus Selector21/1, Bus Selector18/1, Bus Selector6/1, Bus Selector24/1, Bus Selector30/1
  Bus Creator/1 -> Actuators/1
  Bus Selector/1 -> Merge2/1  "<FPC>"
  Bus Selector1/1 -> Merge2/2  "<FPC>"
  Bus Selector2/1 -> Merge2/3  "<FPC>"
  Merge2/1 -> Bus Creator/1  "FPC"
  Merge1/1 -> Bus Creator/2  "SOI"
  Merge5/1 -> Bus Creator/3  "SparkEnergy"
  Merge8/1 -> Bus Creator/5  "Throttle"
  Merge4/1 -> Bus Creator/4  "SparkAdv"
  FuelPump/1(Out) -> Bus Creator/7  "FUELP"
  Bus Selector17/1 -> Merge6/3  "<FuelMult>"
  Bus Selector16/1 -> Merge6/2  "<FuelMult>"
  Bus Selector15/1 -> Merge6/1  "<FuelMult>"
  Merge6/1 -> Bus Creator/8  "FuelMult"
  Bus Selector29/1 -> Merge10/3  "<EOI>"
  Bus Selector28/1 -> Merge10/2  "<EOI>"
  Bus Selector27/1 -> Merge10/1  "<EOI>"
  Merge10/1 -> Bus Creator/6  "MakeUpEOI"
  Hybrid/1 -> Bus Creator/9  "Hybrid"
  Bus Selector8/1 -> Merge3/3  "<EGR>"
  Bus Selector7/1 -> Merge3/2  "<EGR>"
  Bus Selector6/1 -> Merge3/1  "<EGR>"
  Bus Selector26/1 -> Merge9/3  "<OILP>"
  Bus Selector25/1 -> Merge9/2  "<OILP>"
  Bus Selector24/1 -> Merge9/1  "<OILP>"
  Merge3/1 -> Bus Creator/10  "EGR"
  Merge9/1 -> Bus Creator/11  "OILP"
  Merge11/1 -> Bus Creator/12  "O2 Heater"
  Bus Selector30/1 -> Merge11/1  "<O2 Heater>"
  Bus Selector31/1 -> Merge11/2  "<O2 Heater>"
  Bus Selector32/1 -> Merge11/3  "<O2 Heater>"

== system BaseEngineController_A02_bak/Foreground/Control/Controller/Run ==
  Inputs : Inport
  Enable : EnablePort  StatesWhenEnabling=reset  ShowOutputPort=on
  AirFlow Manager : Subsystem
  Base Equiv Ratio : Subsystem  TreatAsAtomicUnit=on
  Bus Creator2 : BusCreator  Inputs=11
  Demux : Demux  Outputs=3
  Documentation_Calibrations_Probes_Block : AutoDoc_lib/Documentation_Calibrations_Probes_Block
  Documentation_Left_Border : AutoDoc_lib/Documentation_Left_Border  layout=Portrait
  Documentation_Right_Border : AutoDoc_lib/Documentation_Right_Border  layout=Portrait
  Documentation_Title_Block : AutoDoc_lib/Documentation_Title_Block
  EGR : Subsystem
  Fuel System Manager : Subsystem  TreatAsAtomicUnit=on
  MaxGovernor : Subsystem  TreatAsAtomicUnit=on
  MinGovernor : Subsystem  TreatAsAtomicUnit=on
  O2 Control : Subsystem  TreatAsAtomicUnit=on
  O2 Heater : Subsystem
  OILP : Constant  OutDataTypeStr=boolean
  ON1 : Constant  OutDataTypeStr=boolean
  Spark Manager : Subsystem  TreatAsAtomicUnit=on
  Zero-Order Hold{1..6,12,16..18} (10 alike) : ZeroOrderHold  SampleTime=-1
  motohawk_replicate1 : MotoHawk_lib/Extra Development Blocks/motohawk_replicate  output_width=8
  motohawk_trigger1 : MotoHawk_lib/Trigger Blocks/motohawk_trigger  Tag=Trigger  trigger=FGND_5XRTI_PERIODIC  function_name=Trigger_FGND_5XRTI_PERIODIC_4476p0004  priority_order=1  inherited=on  run_disabled=off  override_app_monitor=off  target=ECU555_80
  Actuators : Outport
  wires:
  Enable/1 -> O2 Control/1(Reset)
  Inputs/1 -> Spark Manager/2(Sensors), MinGovernor/1(Sensors), MaxGovernor/2(Sensors), AirFlow Manager/3(Sensors), EGR/1(Sensors), O2 Heater/1(Sensors), Base Equiv Ratio/1(Sensors), O2 Control/2(Sensors), Fuel System Manager/1(Sensors)
  ON1/1 -> Zero-Order Hold16/1  "FUELP"
  Bus Creator2/1 -> Actuators/1
  Spark Manager/1(SparkEnergy) -> Zero-Order Hold1/1  "SparkEnergy"
  MinGovernor/1(MinGovInfo) -> Spark Manager/3(RPM_Ctrl_Ofst), MaxGovernor/1(MinGovInfo), AirFlow Manager/1(MinGovInfo)
  Spark Manager/2(SpkAdv) -> Zero-Order Hold12/1  "SparkAdv"
  Base Equiv Ratio/1(EquivRatioInfo) -> O2 Control/3(Desired_Equiv), Spark Manager/1(DesEquivRatio)
  MaxGovernor/1(MaxGovInfo) -> AirFlow Manager/2(MaxGovInfo)
  AirFlow Manager/1(ETC%) -> Zero-Order Hold18/1  "Throttle"
  Fuel System Manager/1(DesiredFuel) -> motohawk_replicate1/1
  Fuel System Manager/2(InjTiming) -> Zero-Order Hold17/1  "SOI"
  Fuel System Manager/3(EOI) -> Zero-Order Hold3/1  "EOI"
  O2 Control/1(O2_Info) -> Fuel System Manager/2(O2_Info)
  motohawk_replicate1/1 -> Bus Creator2/1  "FPC"
  Fuel System Manager/4(FuelMult) -> Zero-Order Hold4/1  "FuelMult"
  Zero-Order Hold1/1 -> Bus Creator2/5  "SparkEnergy"
  Zero-Order Hold12/1 -> Bus Creator2/6  "SparkAdv"
  Zero-Order Hold16/1 -> Bus Creator2/8  "FUELP"
  Zero-Order Hold18/1 -> Bus Creator2/7  "Throttle"
  Zero-Order Hold3/1 -> Bus Creator2/3  "EOI"
  Zero-Order Hold4/1 -> Bus Creator2/4  "FuelMult"
  Zero-Order Hold17/1 -> Bus Creator2/2  "SOI"
  Demux/1 -> MinGovernor/Trigger
  Demux/2 -> MaxGovernor/Trigger
  Demux/3 -> Spark Manager/Trigger
  Zero-Order Hold2/1 -> Bus Creator2/9  "EGR"
  EGR/1(EGR%) -> Zero-Order Hold2/1
  OILP/1 -> Zero-Order Hold5/1  "FUELP"
  Zero-Order Hold5/1 -> Bus Creator2/10  "OILP"
  Zero-Order Hold6/1 -> Bus Creator2/11  "O2 Heater"
  O2 Heater/1(Heater ON) -> Zero-Order Hold6/1
  motohawk_trigger1/1 -> Demux/1
